simulink model "ClimaModMgr"  (R2022b)
settings: Solver=FixedStepDiscrete  StartTime=0.0  StopTime=10.0  FixedStep=0.1  RelTol=1e-3
settings Model: Creator=blue.ge  LastModifiedBy=blue.ge
settings DataIOCC: SaveFormat=StructureWithTime
settings OptimizationCC: DefaultParameterBehavior=Inlined  EnhancedBackFolding=on  PassReuseOutputArgsAs=Individual arguments  ZeroExternalMemoryAtStartup=off  ZeroInternalMemoryAtStartup=off  OptimizeBlockOrder=speed  DifferentSizesBufferReuse=on  OptimizationCustomize=off  EfficientTunableParamExpr=on
settings DebuggingCC: ParameterTunabilityLossMsg=error
settings ModelReferenceCC: ModelReferenceNumInstancesAllowed=Single
settings SFSimCC: MATLABDynamicMemAlloc=off
settings RTWCC: Description=AUTOSAR  SystemTargetFile=autosar.tlc  GenCodeOnly=on  TemplateMakefile=ert_default_tmf
settings CodeAppCC: ForceParamTrailComments=on  IgnoreCustomStorageClasses=off  OperatorAnnotations=on  SimulinkDataObjDesc=on  SFDataObjDesc=on  InsertBlockDesc=on
settings STFCustomTargetCC: TargetLangStandard=C99 (ISO)  MultiwordLength=256  ParMdlRefBuildCompliant=on  CompOptLevelCompliant=on  IncludeMdlTerminateFcn=off  CombineOutputUpdateFcns=on  SuppressErrorStatus=on  ERTCustomFileBanners=on  SupportNonFinite=off  SupportComplex=off  SupportContinuousTime=off  SupportNonInlinedSFcns=off  RemoveResetFunc=on  MATLABClassNameForMDSCustomization=Simulink.SoftwareTarget.ERTCustomization  AutosarCompliant=on  UseToolchainInfoCompliant=on  LookupTableObjectStructAxisOrder=2,1,3,4,...  LUTObjectStructOrderEvenSpacing=Size,Table,Breakpoints  SystemTargetFile=autosar.tlc
settings ERTTargetCC: TargetLangStandard=C99 (ISO)  ConcurrentExecutionCompliant=off  IncludeMdlTerminateFcn=off  SuppressErrorStatus=on  SupportNonFinite=off  SupportComplex=off  ModelStepFunctionPrototypeControlCompliant=off  CPPClassGenCompliant=off  AutosarCompliant=on  LookupTableObjectStructAxisOrder=2,1,3,4,...  LUTObjectStructOrderEvenSpacing=Size,Table,Breakpoints  InlinedParameterPlacement=NonHierarchical  ERTCustomFileTemplate=example_file_process.tlc

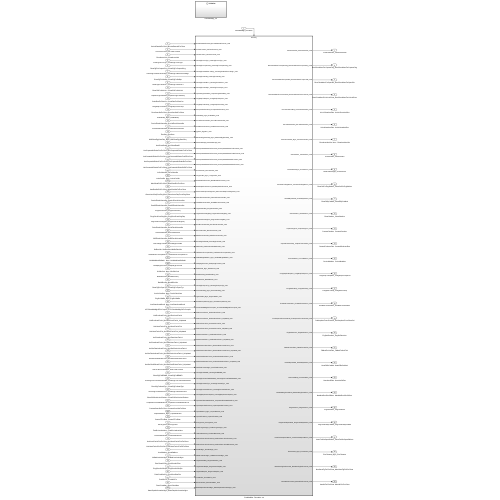
[thumbnail: Simulink's stored preview of the top-level diagram]
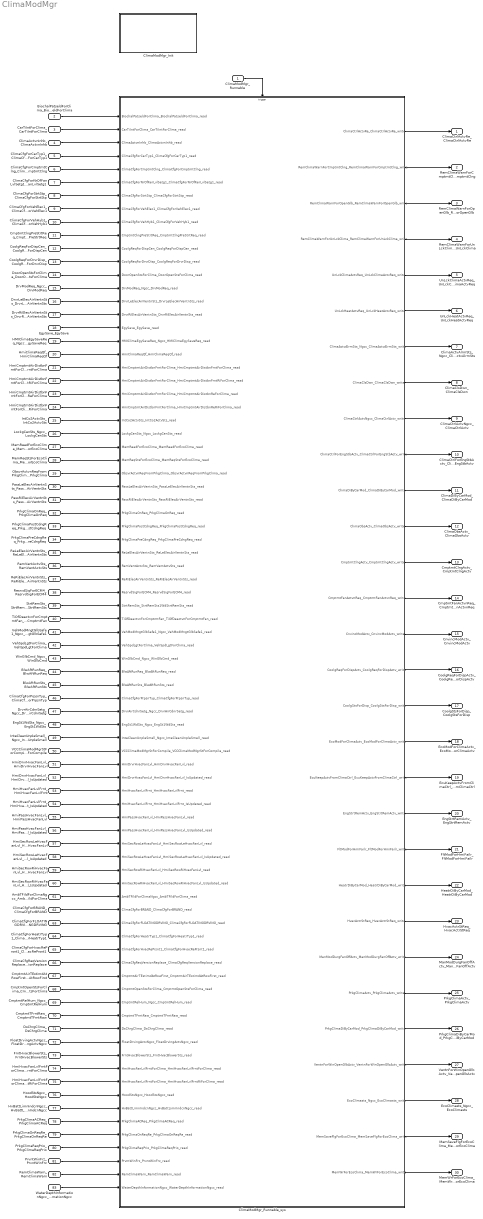
== system ClimaModMgr ==
  ClimaModMgr_Runnable : Inport  SampleTime=0.1  OutputFunctionCall=on
  BiochalPatseldForClima_BiochalPatseldForClima : Inport  Port=2  OutDataTypeStr=Boolean  PortDimensions=1
  CarTiIntForClima_CarTiIntForClima : Inport  Port=3  OutDataTypeStr=CarTiGlb  PortDimensions=1
  ClimaActvnInhb_ClimaActvnInhb : Inport  Port=4  OutDataTypeStr=Enum: OnOff1  PortDimensions=1
  ClimaCfgForCarTyp1_ClimaCfgForCarTyp1 : Inport  Port=5  OutDataTypeStr=Enum: ClimaCfgForCarTyp1  PortDimensions=1
  ClimaCfgForCmptmtClng_ClimaCfgForCmptmtClng : Inport  Port=6  OutDataTypeStr=Boolean  PortDimensions=1
  ClimaCfgForNrOfFanLvlSetg1_ClimaCfgForNrOfFanLvlSetg1 : Inport  Port=7  OutDataTypeStr=Enum: ClimaCfgForNrOfFanLvlSetg1  PortDimensions=1
  ClimaCfgForStrtStp_ClimaCfgForStrtStp : Inport  Port=8  OutDataTypeStr=Enum: CarCfgForStrtStop  PortDimensions=1
  ClimaCfgForVehElec1_ClimaCfgForVehElec1 : Inport  Port=9  OutDataTypeStr=Boolean  PortDimensions=1
  ClimaCfgForVehHyb1_ClimaCfgForVehHyb1 : Inport  Port=10  OutDataTypeStr=Boolean  PortDimensions=1
  CmptmtClngPreStrtReq_CmptmtClngPreStrtReq : Inport  Port=11  OutDataTypeStr=Enum: OnOffNoReq  PortDimensions=1
  CoolgReqForDispCen_CoolgReqForDispCen : Inport  Port=12  OutDataTypeStr=Enum: CoolgReqForDisp  PortDimensions=1
  CoolgReqForDrvrDisp_CoolgReqForDrvrDisp : Inport  Port=13  OutDataTypeStr=Enum: CoolgReqForDisp  PortDimensions=1
  DoorOpenStsForClima_DoorOpenStsForClima : Inport  Port=14  OutDataTypeStr=Boolean  PortDimensions=1
  DrvModReq_Ngcc_DrvModReq : Inport  Port=15  OutDataTypeStr=Enum: DrvModReqType1  PortDimensions=1
  DrvrLeElecAirVentnSts_DrvrLeElecAirVentnSts : Inport  Port=16  OutDataTypeStr=Enum: OnOff1  PortDimensions=1
  DrvrRiElecAirVentnSts_DrvrRiElecAirVentnSts : Inport  Port=17  OutDataTypeStr=Enum: OnOff1  PortDimensions=1
  EgySave_EgySave : Inport  Port=18  OutDataTypeStr=Enum: OnOff1  PortDimensions=1
  HMIClimaEgySaveReq_Ngcc_HMIClimaEgySaveReq : Inport  Port=19  OutDataTypeStr=Enum: VentnActr01BlckInd  PortDimensions=1
  HmiClimaReqQf_HmiClimaReqQf : Inport  Port=20  OutDataTypeStr=Enum: HmiClimaReqQf  PortDimensions=1
  HmiCmptmtAirDistbnFrntForClima_HmiCmptmtAirDistbnFrntForClima : Inport  Port=21  OutDataTypeStr=Enum: HmiCmptmtAirDistbnFrnt  PortDimensions=1
  HmiCmptmtAirDistbnFrntForClima_HmiCmptmtAirDistbnFrntRiForClima : Inport  Port=22  OutDataTypeStr=Enum: HmiCmptmtAirDistbnFrnt  PortDimensions=1
  HmiCmptmtAirDistbnFrntForClima_HmiCmptmtAirDistbnReForClima : Inport  Port=23  OutDataTypeStr=Enum: HmiCmptmtAirDistbnFrnt  PortDimensions=1
  HmiCmptmtAirDistbnFrntForClima_HmiCmptmtAirDistbnReRiForClima : Inport  Port=24  OutDataTypeStr=Enum: HmiCmptmtAirDistbnFrnt  PortDimensions=1
  IntCo2ActvSts_IntCo2ActvSts : Inport  Port=25  OutDataTypeStr=Enum: IntCo2Sts  PortDimensions=1
  LockgCenSts_Ngcc_LockgCenSts : Inport  Port=26  OutDataTypeStr=Bus: LockgCenSts3  PortDimensions=1  BusOutputAsStruct=on
  MemReadForEcoClima_MemReadForEcoClima : Inport  Port=27  OutDataTypeStr=Bus: MemWrForEcoClima  PortDimensions=1  BusOutputAsStruct=on
  MemReqStsForEcoClima_MemReqStsForEcoClima : Inport  Port=28  OutDataTypeStr=Enum: MemReqSts  PortDimensions=1
  ObsvrActvnReqFromPrkgClima_ObsvrActvnReqFromPrkgClima : Inport  Port=29  OutDataTypeStr=Boolean  PortDimensions=1
  PassLeElecAirVentnSts_PassLeElecAirVentnSts : Inport  Port=30  OutDataTypeStr=Enum: OnOff1  PortDimensions=1
  PassRiElecAirVentnSts_PassRiElecAirVentnSts : Inport  Port=31  OutDataTypeStr=Enum: OnOff1  PortDimensions=1
  PrkgClimaOnReq_PrkgClimaOnReq : Inport  Port=32  OutDataTypeStr=Boolean  PortDimensions=1
  PrkgClimaPostCdngReq_PrkgClimaPostCdngReq : Inport  Port=33  OutDataTypeStr=Boolean  PortDimensions=1
  PrkgClimaPreCdngReq_PrkgClimaPreCdngReq : Inport  Port=34  OutDataTypeStr=Boolean  PortDimensions=1
  ReLeElecAirVentnSts_ReLeElecAirVentnSts : Inport  Port=35  OutDataTypeStr=Enum: OnOff1  PortDimensions=1
  RemVentActvSts_RemVentActvSts : Inport  Port=36  OutDataTypeStr=Enum: OnOff1  PortDimensions=1
  ReRiElecAirVentnSts_ReRiElecAirVentnSts : Inport  Port=37  OutDataTypeStr=Enum: OnOff1  PortDimensions=1
  ResrvdSigForECM4_ResrvdSigForECM4 : Inport  Port=38  OutDataTypeStr=BattSnsrChksTbl  PortDimensions=1
  StrtRemSts_StrtRemSts1WdStrtRemSts : Inport  Port=39  OutDataTypeStr=Enum: StrtRemSts1  PortDimensions=1
  TiOfDeactvnForCmptmtFan_TiOfDeactvnForCmptmtFan : Inport  Port=40  OutDataTypeStr=Ti33  PortDimensions=1
  VehModMngtGlbSafe1_Ngcc_VehModMngtGlbSafe1 : Inport  Port=41  OutDataTypeStr=Bus: VehModMngtGlbSafe1  PortDimensions=1  BusOutputAsStruct=on
  VehSpdLgtForClima_VehSpdLgtForClima : Inport  Port=42  OutDataTypeStr=Bus: VehSpdLgtForClima  PortDimensions=1  BusOutputAsStruct=on
  WinGlbCmd_Ngcc_WinGlbCmd : Inport  Port=43  OutDataTypeStr=Enum: OpenClsGlbCmd  PortDimensions=1
  BlwAftRunReq_BlwAftRunReq : Inport  Port=44  OutDataTypeStr=Boolean  PortDimensions=1
  BlwAftRunSts_BlwAftRunSts : Inport  Port=45  OutDataTypeStr=Boolean  PortDimensions=1
  ClimaCfgForPrpsnTyp_ClimaCfgForPrpsnTyp : Inport  Port=46  OutDataTypeStr=Enum: CarCfgForPrpsnTypGly  PortDimensions=1
  DrvrAirCdnrSetg_Ngcc_DrvrAirCdnrSetg : Inport  Port=47  OutDataTypeStr=Bus: DrvrAirCdnrSetg  PortDimensions=1  BusOutputAsStruct=on
  EngSt1WdSts_Ngcc_EngSt1WdSts : Inport  Port=48  OutDataTypeStr=Enum: EngSt1  PortDimensions=1
  InteCleanUnpleSmell_Ngcc_InteCleanUnpleSmell : Inport  Port=49  OutDataTypeStr=Enum: OnOff1  PortDimensions=1
  VCCClimaModMgrStForCompile_VCCClimaModMgrStForCompile : Inport  Port=50  OutDataTypeStr=Enum: VCCClimaModMgrSt  PortDimensions=1
  HmiDrvrHvacFanLvl_HmiDrvrHvacFanLvl : Inport  Port=51  OutDataTypeStr=Enum: HmiHvacFanLvl  PortDimensions=1
  HmiDrvrHvacFanLvl_HmiDrvrHvacFanLvl_IsUpdated : Inport  Port=52  OutDataTypeStr=boolean  PortDimensions=1
  HmiHvacFanLvlFrnt_HmiHvacFanLvlFrnt : Inport  Port=53  OutDataTypeStr=Enum: HmiHvacFanLvl  PortDimensions=1
  HmiHvacFanLvlFrnt_HmiHvacFanLvlFrnt_IsUpdated : Inport  Port=54  OutDataTypeStr=boolean  PortDimensions=1
  HmiPassHvacFanLvl_HmiPassHvacFanLvl : Inport  Port=55  OutDataTypeStr=Enum: HmiHvacFanLvl  PortDimensions=1
  HmiPassHvacFanLvl_HmiPassHvacFanLvl_IsUpdated : Inport  Port=56  OutDataTypeStr=boolean  PortDimensions=1
  HmiSecRowLeHvacFanLvl_HmiSecRowLeHvacFanLvl : Inport  Port=57  OutDataTypeStr=Enum: HmiHvacFanLvl  PortDimensions=1
  HmiSecRowLeHvacFanLvl_HmiSecRowLeHvacFanLvl_IsUpdated : Inport  Port=58  OutDataTypeStr=boolean  PortDimensions=1
  HmiSecRowRiHvacFanLvl_HmiSecRowRiHvacFanLvl : Inport  Port=59  OutDataTypeStr=Enum: HmiHvacFanLvl  PortDimensions=1
  HmiSecRowRiHvacFanLvl_HmiSecRowRiHvacFanLvl_IsUpdated : Inport  Port=60  OutDataTypeStr=boolean  PortDimensions=1
  AmbTFildForClimaNgcc_AmbTFildForClima : Inport  Port=61  OutDataTypeStr=T30  PortDimensions=1
  ClimaCfgForBRAND_ClimaCfgForBRAND : Inport  Port=62  OutDataTypeStr=Enum: ClimaCfgForBRAND  PortDimensions=1
  ClimaCfgForFLOATINGDRVING_ClimaCfgForFLOATINGDRVING : Inport  Port=63  OutDataTypeStr=Enum: FLOATINGDRVING  PortDimensions=1
  ClimaCfgForHeatrTyp1_ClimaCfgForHeatrTyp1 : Inport  Port=64  OutDataTypeStr=Enum: ClimaCfgForHeatrTyp1  PortDimensions=1
  ClimaCfgForHvacRePrsnt1_ClimaCfgForHvacRePrsnt1 : Inport  Port=65  OutDataTypeStr=Enum: CarCfgForHvacRe  PortDimensions=1
  ClimaCfgReqVersionReplace_ClimaCfgReqVersionReplace : Inport  Port=66  OutDataTypeStr=UInt8  PortDimensions=1
  CmptmtAirTEstimdAtRowFirst_CmptmtAirTEstimdAtRowFirst : Inport  Port=67  OutDataTypeStr=T30  PortDimensions=1
  CmptmtOpenStsForClima_CmptmtOpenStsForClima : Inport  Port=68  OutDataTypeStr=Boolean  PortDimensions=1
  CmptmtRelHum_Ngcc_CmptmtRelHum : Inport  Port=69  OutDataTypeStr=Bus: CmptmRelHum  PortDimensions=1  BusOutputAsStruct=on
  CmptmtTFrntRaw_CmptmtTFrntRaw : Inport  Port=70  OutDataTypeStr=Bus: CmptmtTFrnt  PortDimensions=1  BusOutputAsStruct=on
  DsChrgClima_DsChrgClima : Inport  Port=71  OutDataTypeStr=Boolean  PortDimensions=1
  FloatDrvingActvNgcc_FloatDrvingActvNgcc : Inport  Port=72  OutDataTypeStr=Bus: FloatDrvingActv  PortDimensions=1  BusOutputAsStruct=on
  FrntHvacBlowerSts_FrntHvacBlowerSts : Inport  Port=73  OutDataTypeStr=Enum: HvacFanSts  PortDimensions=1
  HmiHvacFanLvlFrntForClima_HmiHvacFanLvlFrntForClima : Inport  Port=74  OutDataTypeStr=Enum: HmiHvacFanLvl  PortDimensions=1
  HmiHvacFanLvlFrntForClima_HmiHvacFanLvlFrntRiForClima : Inport  Port=75  OutDataTypeStr=Enum: HmiHvacFanLvl  PortDimensions=1
  HoodStsNgcc_HoodStsNgcc : Inport  Port=76  OutDataTypeStr=Enum: DoorSts2  PortDimensions=1
  HvBattLimnIndcnNgcc_HvBattLimnIndcnNgcc : Inport  Port=77  OutDataTypeStr=UInt16  PortDimensions=1
  PrkgClimaACReq_PrkgClimaACReq : Inport  Port=78  OutDataTypeStr=Enum: HmiCmptmtCoolgReq  PortDimensions=1
  PrkgClimaOnReqRe_PrkgClimaOnReqRe : Inport  Port=79  OutDataTypeStr=Enum: OnOff1  PortDimensions=1
  PrkgClimaReqPrio_PrkgClimaReqPrio : Inport  Port=80  OutDataTypeStr=Boolean  PortDimensions=1
  PrvntWinFrz_PrvntWinFrz : Inport  Port=81  OutDataTypeStr=Boolean  PortDimensions=1
  RemClimaWarn_RemClimaWarn : Inport  Port=82  OutDataTypeStr=Enum: ClimaWarn  PortDimensions=1
  WaterDepthInformationNgcc_WaterDepthInformationNgcc : Inport  Port=83  OutDataTypeStr=Bus: WaterDepthInformation  PortDimensions=1  BusOutputAsStruct=on
  ClimaModMgr_Init : Subsystem
  ClimaModMgr_Runnable_sys : Subsystem
  ClimaCtrlActvRe_ClimaCtrlActvRe : Outport  OutDataTypeStr=Boolean  PortDimensions=1
  RemClimaWarnForCmptmtClng_RemClimaWarnForCmptmtClng : Outport  Port=2  OutDataTypeStr=Enum: ClimaWarn  PortDimensions=1
  RemClimaWarnForOpenGlb_RemClimaWarnForOpenGlb : Outport  Port=3  OutDataTypeStr=Enum: ClimaWarn  PortDimensions=1
  RemClimaWarnForUnLckClima_RemClimaWarnForUnLckClima : Outport  Port=4  OutDataTypeStr=Enum: ClimaWarn  PortDimensions=1
  UnLckClimaActvReq_UnLckClimaActvReq : Outport  Port=5  OutDataTypeStr=Enum: UnLckCoolActvLvl  PortDimensions=1
  UnLckHeatActvReq_UnLckHeatActvReq : Outport  Port=6  OutDataTypeStr=Enum: UnLckHeatActvLvl  PortDimensions=1
  ClimaActvAlrmSts_Ngcc_ClimaActvAlrmSts : Outport  Port=7  OutDataTypeStr=Enum: OnOff1  PortDimensions=1
  ClimaClsDwn_ClimaClsDwn : Outport  Port=8  OutDataTypeStr=Boolean  PortDimensions=1
  ClimaCtrlActvNgcc_ClimaCtrlActv : Outport  Port=9  OutDataTypeStr=Boolean  PortDimensions=1
  ClimaCtrlForEngStbActv_ClimaCtrlForEngStbActv : Outport  Port=10  OutDataTypeStr=Boolean  PortDimensions=1
  ClimaDiByCarMod_ClimaDiByCarMod : Outport  Port=11  OutDataTypeStr=Boolean  PortDimensions=1
  ClimaObsActv_ClimaObsActv : Outport  Port=12  OutDataTypeStr=Boolean  PortDimensions=1
  CmptmtClngActv_CmptmtClngActv : Outport  Port=13  OutDataTypeStr=Enum: CmptmtClngActv  PortDimensions=1
  CmptmtFanActvnReq_CmptmtFanActvnReq : Outport  Port=14  OutDataTypeStr=Enum: OnOff1  PortDimensions=1
  CnvincModActv_CnvincModActv : Outport  Port=15  OutDataTypeStr=Boolean  PortDimensions=1
  CoolgReqForDispActv_CoolgReqForDispActv : Outport  Port=16  OutDataTypeStr=Enum: CoolgReqForDisp  PortDimensions=1
  CoolgStsForDisp_CoolgStsForDisp : Outport  Port=17  OutDataTypeStr=Enum: CoolgStsForDisp  PortDimensions=1
  EcoModForClimaActv_EcoModForClimaActv : Outport  Port=18  OutDataTypeStr=Enum: OnOff1  PortDimensions=1
  EcuKeepActvFromClimaCtrl_EcuKeepActvFromClimaCtrl : Outport  Port=19  OutDataTypeStr=Enum: OnOff1  PortDimensions=1
  EngStrtRemActv_EngStrtRemActv : Outport  Port=20  OutDataTypeStr=Boolean  PortDimensions=1
  FltModForHmiFailr_FltModForHmiFailr : Outport  Port=21  OutDataTypeStr=Boolean  PortDimensions=1
  HeatrDiByCarMod_HeatrDiByCarMod : Outport  Port=22  OutDataTypeStr=Boolean  PortDimensions=1
  HvacActrStReq_HvacActrStReq : Outport  Port=23  OutDataTypeStr=Enum: HvacActrSt  PortDimensions=1
  ManModDurgFanOffActv_ManModDurgFanOffActv : Outport  Port=24  OutDataTypeStr=Boolean  PortDimensions=1
  PrkgClimaActv_PrkgClimaActv : Outport  Port=25  OutDataTypeStr=Enum: PrkgClimaActv  PortDimensions=1
  PrkgClimaDiByCarMod_PrkgClimaDiByCarMod : Outport  Port=26  OutDataTypeStr=Boolean  PortDimensions=1
  VentnForWinOpenGlbActv_VentnForWinOpenGlbActv : Outport  Port=27  OutDataTypeStr=Boolean  PortDimensions=1
  EcoClimasts_Ngcc_EcoClimasts : Outport  Port=28  OutDataTypeStr=Enum: OnOff1  PortDimensions=1
  MemSaveFlgForEcoClima_MemSaveFlgForEcoClima : Outport  Port=29  OutDataTypeStr=Boolean  PortDimensions=1
  MemWrForEcoClima_MemWrForEcoClima : Outport  Port=30  OutDataTypeStr=Bus: MemWrForEcoClima  PortDimensions=1  BusOutputAsStruct=on
  wires:
  BiochalPatseldForClima_BiochalPatseldForClima/1 -> ClimaModMgr_Runnable_sys/1(BiochalPatseldForClima_BiochalPatseldForClima_read)
  CarTiIntForClima_CarTiIntForClima/1 -> ClimaModMgr_Runnable_sys/2(CarTiIntForClima_CarTiIntForClima_read)
  ClimaActvnInhb_ClimaActvnInhb/1 -> ClimaModMgr_Runnable_sys/3(ClimaActvnInhb_ClimaActvnInhb_read)
  ClimaCfgForCarTyp1_ClimaCfgForCarTyp1/1 -> ClimaModMgr_Runnable_sys/4(ClimaCfgForCarTyp1_ClimaCfgForCarTyp1_read)
  ClimaCfgForCmptmtClng_ClimaCfgForCmptmtClng/1 -> ClimaModMgr_Runnable_sys/5(ClimaCfgForCmptmtClng_ClimaCfgForCmptmtClng_read)
  ClimaCfgForNrOfFanLvlSetg1_ClimaCfgForNrOfFanLvlSetg1/1 -> ClimaModMgr_Runnable_sys/6(ClimaCfgForNrOfFanLvlSetg1_ClimaCfgForNrOfFanLvlSetg1_read)
  ClimaCfgForStrtStp_ClimaCfgForStrtStp/1 -> ClimaModMgr_Runnable_sys/7(ClimaCfgForStrtStp_ClimaCfgForStrtStp_read)
  ClimaCfgForVehElec1_ClimaCfgForVehElec1/1 -> ClimaModMgr_Runnable_sys/8(ClimaCfgForVehElec1_ClimaCfgForVehElec1_read)
  ClimaCfgForVehHyb1_ClimaCfgForVehHyb1/1 -> ClimaModMgr_Runnable_sys/9(ClimaCfgForVehHyb1_ClimaCfgForVehHyb1_read)
  CmptmtClngPreStrtReq_CmptmtClngPreStrtReq/1 -> ClimaModMgr_Runnable_sys/10(CmptmtClngPreStrtReq_CmptmtClngPreStrtReq_read)
  CoolgReqForDispCen_CoolgReqForDispCen/1 -> ClimaModMgr_Runnable_sys/11(CoolgReqForDispCen_CoolgReqForDispCen_read)
  CoolgReqForDrvrDisp_CoolgReqForDrvrDisp/1 -> ClimaModMgr_Runnable_sys/12(CoolgReqForDrvrDisp_CoolgReqForDrvrDisp_read)
  DoorOpenStsForClima_DoorOpenStsForClima/1 -> ClimaModMgr_Runnable_sys/13(DoorOpenStsForClima_DoorOpenStsForClima_read)
  DrvModReq_Ngcc_DrvModReq/1 -> ClimaModMgr_Runnable_sys/14(DrvModReq_Ngcc_DrvModReq_read)
  DrvrLeElecAirVentnSts_DrvrLeElecAirVentnSts/1 -> ClimaModMgr_Runnable_sys/15(DrvrLeElecAirVentnSts_DrvrLeElecAirVentnSts_read)
  DrvrRiElecAirVentnSts_DrvrRiElecAirVentnSts/1 -> ClimaModMgr_Runnable_sys/16(DrvrRiElecAirVentnSts_DrvrRiElecAirVentnSts_read)
  EgySave_EgySave/1 -> ClimaModMgr_Runnable_sys/17(EgySave_EgySave_read)
  HMIClimaEgySaveReq_Ngcc_HMIClimaEgySaveReq/1 -> ClimaModMgr_Runnable_sys/18(HMIClimaEgySaveReq_Ngcc_HMIClimaEgySaveReq_read)
  HmiClimaReqQf_HmiClimaReqQf/1 -> ClimaModMgr_Runnable_sys/19(HmiClimaReqQf_HmiClimaReqQf_read)
  HmiCmptmtAirDistbnFrntForClima_HmiCmptmtAirDistbnFrntForClima/1 -> ClimaModMgr_Runnable_sys/20(HmiCmptmtAirDistbnFrntForClima_HmiCmptmtAirDistbnFrntForClima_read)
  HmiCmptmtAirDistbnFrntForClima_HmiCmptmtAirDistbnFrntRiForClima/1 -> ClimaModMgr_Runnable_sys/21(HmiCmptmtAirDistbnFrntForClima_HmiCmptmtAirDistbnFrntRiForClima_read)
  HmiCmptmtAirDistbnFrntForClima_HmiCmptmtAirDistbnReForClima/1 -> ClimaModMgr_Runnable_sys/22(HmiCmptmtAirDistbnFrntForClima_HmiCmptmtAirDistbnReForClima_read)
  HmiCmptmtAirDistbnFrntForClima_HmiCmptmtAirDistbnReRiForClima/1 -> ClimaModMgr_Runnable_sys/23(HmiCmptmtAirDistbnFrntForClima_HmiCmptmtAirDistbnReRiForClima_read)
  IntCo2ActvSts_IntCo2ActvSts/1 -> ClimaModMgr_Runnable_sys/24(IntCo2ActvSts_IntCo2ActvSts_read)
  LockgCenSts_Ngcc_LockgCenSts/1 -> ClimaModMgr_Runnable_sys/25(LockgCenSts_Ngcc_LockgCenSts_read)
  MemReadForEcoClima_MemReadForEcoClima/1 -> ClimaModMgr_Runnable_sys/26(MemReadForEcoClima_MemReadForEcoClima_read)
  MemReqStsForEcoClima_MemReqStsForEcoClima/1 -> ClimaModMgr_Runnable_sys/27(MemReqStsForEcoClima_MemReqStsForEcoClima_read)
  ObsvrActvnReqFromPrkgClima_ObsvrActvnReqFromPrkgClima/1 -> ClimaModMgr_Runnable_sys/28(ObsvrActvnReqFromPrkgClima_ObsvrActvnReqFromPrkgClima_read)
  PassLeElecAirVentnSts_PassLeElecAirVentnSts/1 -> ClimaModMgr_Runnable_sys/29(PassLeElecAirVentnSts_PassLeElecAirVentnSts_read)
  PassRiElecAirVentnSts_PassRiElecAirVentnSts/1 -> ClimaModMgr_Runnable_sys/30(PassRiElecAirVentnSts_PassRiElecAirVentnSts_read)
  PrkgClimaOnReq_PrkgClimaOnReq/1 -> ClimaModMgr_Runnable_sys/31(PrkgClimaOnReq_PrkgClimaOnReq_read)
  PrkgClimaPostCdngReq_PrkgClimaPostCdngReq/1 -> ClimaModMgr_Runnable_sys/32(PrkgClimaPostCdngReq_PrkgClimaPostCdngReq_read)
  PrkgClimaPreCdngReq_PrkgClimaPreCdngReq/1 -> ClimaModMgr_Runnable_sys/33(PrkgClimaPreCdngReq_PrkgClimaPreCdngReq_read)
  ReLeElecAirVentnSts_ReLeElecAirVentnSts/1 -> ClimaModMgr_Runnable_sys/34(ReLeElecAirVentnSts_ReLeElecAirVentnSts_read)
  RemVentActvSts_RemVentActvSts/1 -> ClimaModMgr_Runnable_sys/35(RemVentActvSts_RemVentActvSts_read)
  ReRiElecAirVentnSts_ReRiElecAirVentnSts/1 -> ClimaModMgr_Runnable_sys/36(ReRiElecAirVentnSts_ReRiElecAirVentnSts_read)
  ResrvdSigForECM4_ResrvdSigForECM4/1 -> ClimaModMgr_Runnable_sys/37(ResrvdSigForECM4_ResrvdSigForECM4_read)
  StrtRemSts_StrtRemSts1WdStrtRemSts/1 -> ClimaModMgr_Runnable_sys/38(StrtRemSts_StrtRemSts1WdStrtRemSts_read)
  TiOfDeactvnForCmptmtFan_TiOfDeactvnForCmptmtFan/1 -> ClimaModMgr_Runnable_sys/39(TiOfDeactvnForCmptmtFan_TiOfDeactvnForCmptmtFan_read)
  VehModMngtGlbSafe1_Ngcc_VehModMngtGlbSafe1/1 -> ClimaModMgr_Runnable_sys/40(VehModMngtGlbSafe1_Ngcc_VehModMngtGlbSafe1_read)
  VehSpdLgtForClima_VehSpdLgtForClima/1 -> ClimaModMgr_Runnable_sys/41(VehSpdLgtForClima_VehSpdLgtForClima_read)
  WinGlbCmd_Ngcc_WinGlbCmd/1 -> ClimaModMgr_Runnable_sys/42(WinGlbCmd_Ngcc_WinGlbCmd_read)
  BlwAftRunReq_BlwAftRunReq/1 -> ClimaModMgr_Runnable_sys/43(BlwAftRunReq_BlwAftRunReq_read)
  BlwAftRunSts_BlwAftRunSts/1 -> ClimaModMgr_Runnable_sys/44(BlwAftRunSts_BlwAftRunSts_read)
  ClimaCfgForPrpsnTyp_ClimaCfgForPrpsnTyp/1 -> ClimaModMgr_Runnable_sys/45(ClimaCfgForPrpsnTyp_ClimaCfgForPrpsnTyp_read)
  DrvrAirCdnrSetg_Ngcc_DrvrAirCdnrSetg/1 -> ClimaModMgr_Runnable_sys/46(DrvrAirCdnrSetg_Ngcc_DrvrAirCdnrSetg_read)
  EngSt1WdSts_Ngcc_EngSt1WdSts/1 -> ClimaModMgr_Runnable_sys/47(EngSt1WdSts_Ngcc_EngSt1WdSts_read)
  InteCleanUnpleSmell_Ngcc_InteCleanUnpleSmell/1 -> ClimaModMgr_Runnable_sys/48(InteCleanUnpleSmell_Ngcc_InteCleanUnpleSmell_read)
  VCCClimaModMgrStForCompile_VCCClimaModMgrStForCompile/1 -> ClimaModMgr_Runnable_sys/49(VCCClimaModMgrStForCompile_VCCClimaModMgrStForCompile_read)
  HmiDrvrHvacFanLvl_HmiDrvrHvacFanLvl/1 -> ClimaModMgr_Runnable_sys/50(HmiDrvrHvacFanLvl_HmiDrvrHvacFanLvl_read)
  HmiDrvrHvacFanLvl_HmiDrvrHvacFanLvl_IsUpdated/1 -> ClimaModMgr_Runnable_sys/51(HmiDrvrHvacFanLvl_HmiDrvrHvacFanLvl_IsUpdated_read)
  HmiHvacFanLvlFrnt_HmiHvacFanLvlFrnt/1 -> ClimaModMgr_Runnable_sys/52(HmiHvacFanLvlFrnt_HmiHvacFanLvlFrnt_read)
  HmiHvacFanLvlFrnt_HmiHvacFanLvlFrnt_IsUpdated/1 -> ClimaModMgr_Runnable_sys/53(HmiHvacFanLvlFrnt_HmiHvacFanLvlFrnt_IsUpdated_read)
  HmiPassHvacFanLvl_HmiPassHvacFanLvl/1 -> ClimaModMgr_Runnable_sys/54(HmiPassHvacFanLvl_HmiPassHvacFanLvl_read)
  HmiPassHvacFanLvl_HmiPassHvacFanLvl_IsUpdated/1 -> ClimaModMgr_Runnable_sys/55(HmiPassHvacFanLvl_HmiPassHvacFanLvl_IsUpdated_read)
  HmiSecRowLeHvacFanLvl_HmiSecRowLeHvacFanLvl/1 -> ClimaModMgr_Runnable_sys/56(HmiSecRowLeHvacFanLvl_HmiSecRowLeHvacFanLvl_read)
  HmiSecRowLeHvacFanLvl_HmiSecRowLeHvacFanLvl_IsUpdated/1 -> ClimaModMgr_Runnable_sys/57(HmiSecRowLeHvacFanLvl_HmiSecRowLeHvacFanLvl_IsUpdated_read)
  HmiSecRowRiHvacFanLvl_HmiSecRowRiHvacFanLvl/1 -> ClimaModMgr_Runnable_sys/58(HmiSecRowRiHvacFanLvl_HmiSecRowRiHvacFanLvl_read)
  HmiSecRowRiHvacFanLvl_HmiSecRowRiHvacFanLvl_IsUpdated/1 -> ClimaModMgr_Runnable_sys/59(HmiSecRowRiHvacFanLvl_HmiSecRowRiHvacFanLvl_IsUpdated_read)
  AmbTFildForClimaNgcc_AmbTFildForClima/1 -> ClimaModMgr_Runnable_sys/60(AmbTFildForClimaNgcc_AmbTFildForClima_read)
  ClimaCfgForBRAND_ClimaCfgForBRAND/1 -> ClimaModMgr_Runnable_sys/61(ClimaCfgForBRAND_ClimaCfgForBRAND_read)
  ClimaCfgForFLOATINGDRVING_ClimaCfgForFLOATINGDRVING/1 -> ClimaModMgr_Runnable_sys/62(ClimaCfgForFLOATINGDRVING_ClimaCfgForFLOATINGDRVING_read)
  ClimaCfgForHeatrTyp1_ClimaCfgForHeatrTyp1/1 -> ClimaModMgr_Runnable_sys/63(ClimaCfgForHeatrTyp1_ClimaCfgForHeatrTyp1_read)
  ClimaCfgForHvacRePrsnt1_ClimaCfgForHvacRePrsnt1/1 -> ClimaModMgr_Runnable_sys/64(ClimaCfgForHvacRePrsnt1_ClimaCfgForHvacRePrsnt1_read)
  ClimaCfgReqVersionReplace_ClimaCfgReqVersionReplace/1 -> ClimaModMgr_Runnable_sys/65(ClimaCfgReqVersionReplace_ClimaCfgReqVersionReplace_read)
  CmptmtAirTEstimdAtRowFirst_CmptmtAirTEstimdAtRowFirst/1 -> ClimaModMgr_Runnable_sys/66(CmptmtAirTEstimdAtRowFirst_CmptmtAirTEstimdAtRowFirst_read)
  CmptmtOpenStsForClima_CmptmtOpenStsForClima/1 -> ClimaModMgr_Runnable_sys/67(CmptmtOpenStsForClima_CmptmtOpenStsForClima_read)
  CmptmtRelHum_Ngcc_CmptmtRelHum/1 -> ClimaModMgr_Runnable_sys/68(CmptmtRelHum_Ngcc_CmptmtRelHum_read)
  CmptmtTFrntRaw_CmptmtTFrntRaw/1 -> ClimaModMgr_Runnable_sys/69(CmptmtTFrntRaw_CmptmtTFrntRaw_read)
  DsChrgClima_DsChrgClima/1 -> ClimaModMgr_Runnable_sys/70(DsChrgClima_DsChrgClima_read)
  FloatDrvingActvNgcc_FloatDrvingActvNgcc/1 -> ClimaModMgr_Runnable_sys/71(FloatDrvingActvNgcc_FloatDrvingActvNgcc_read)
  FrntHvacBlowerSts_FrntHvacBlowerSts/1 -> ClimaModMgr_Runnable_sys/72(FrntHvacBlowerSts_FrntHvacBlowerSts_read)
  HmiHvacFanLvlFrntForClima_HmiHvacFanLvlFrntForClima/1 -> ClimaModMgr_Runnable_sys/73(HmiHvacFanLvlFrntForClima_HmiHvacFanLvlFrntForClima_read)
  HmiHvacFanLvlFrntForClima_HmiHvacFanLvlFrntRiForClima/1 -> ClimaModMgr_Runnable_sys/74(HmiHvacFanLvlFrntForClima_HmiHvacFanLvlFrntRiForClima_read)
  HoodStsNgcc_HoodStsNgcc/1 -> ClimaModMgr_Runnable_sys/75(HoodStsNgcc_HoodStsNgcc_read)
  HvBattLimnIndcnNgcc_HvBattLimnIndcnNgcc/1 -> ClimaModMgr_Runnable_sys/76(HvBattLimnIndcnNgcc_HvBattLimnIndcnNgcc_read)
  PrkgClimaACReq_PrkgClimaACReq/1 -> ClimaModMgr_Runnable_sys/77(PrkgClimaACReq_PrkgClimaACReq_read)
  PrkgClimaOnReqRe_PrkgClimaOnReqRe/1 -> ClimaModMgr_Runnable_sys/78(PrkgClimaOnReqRe_PrkgClimaOnReqRe_read)
  PrkgClimaReqPrio_PrkgClimaReqPrio/1 -> ClimaModMgr_Runnable_sys/79(PrkgClimaReqPrio_PrkgClimaReqPrio_read)
  PrvntWinFrz_PrvntWinFrz/1 -> ClimaModMgr_Runnable_sys/80(PrvntWinFrz_PrvntWinFrz_read)
  RemClimaWarn_RemClimaWarn/1 -> ClimaModMgr_Runnable_sys/81(RemClimaWarn_RemClimaWarn_read)
  WaterDepthInformationNgcc_WaterDepthInformationNgcc/1 -> ClimaModMgr_Runnable_sys/82(WaterDepthInformationNgcc_WaterDepthInformationNgcc_read)
  ClimaModMgr_Runnable_sys/1(ClimaCtrlActvRe_ClimaCtrlActvRe_write) -> ClimaCtrlActvRe_ClimaCtrlActvRe/1
  ClimaModMgr_Runnable_sys/2(RemClimaWarnForCmptmtClng_RemClimaWarnForCmptmtClng_write) -> RemClimaWarnForCmptmtClng_RemClimaWarnForCmptmtClng/1
  ClimaModMgr_Runnable_sys/3(RemClimaWarnForOpenGlb_RemClimaWarnForOpenGlb_write) -> RemClimaWarnForOpenGlb_RemClimaWarnForOpenGlb/1
  ClimaModMgr_Runnable_sys/4(RemClimaWarnForUnLckClima_RemClimaWarnForUnLckClima_write) -> RemClimaWarnForUnLckClima_RemClimaWarnForUnLckClima/1
  ClimaModMgr_Runnable_sys/5(UnLckClimaActvReq_UnLckClimaActvReq_write) -> UnLckClimaActvReq_UnLckClimaActvReq/1
  ClimaModMgr_Runnable_sys/6(UnLckHeatActvReq_UnLckHeatActvReq_write) -> UnLckHeatActvReq_UnLckHeatActvReq/1
  ClimaModMgr_Runnable_sys/7(ClimaActvAlrmSts_Ngcc_ClimaActvAlrmSts_write) -> ClimaActvAlrmSts_Ngcc_ClimaActvAlrmSts/1
  ClimaModMgr_Runnable_sys/8(ClimaClsDwn_ClimaClsDwn_write) -> ClimaClsDwn_ClimaClsDwn/1
  ClimaModMgr_Runnable_sys/9(ClimaCtrlActvNgcc_ClimaCtrlActv_write) -> ClimaCtrlActvNgcc_ClimaCtrlActv/1
  ClimaModMgr_Runnable_sys/10(ClimaCtrlForEngStbActv_ClimaCtrlForEngStbActv_write) -> ClimaCtrlForEngStbActv_ClimaCtrlForEngStbActv/1
  ClimaModMgr_Runnable_sys/11(ClimaDiByCarMod_ClimaDiByCarMod_write) -> ClimaDiByCarMod_ClimaDiByCarMod/1
  ClimaModMgr_Runnable_sys/12(ClimaObsActv_ClimaObsActv_write) -> ClimaObsActv_ClimaObsActv/1
  ClimaModMgr_Runnable_sys/13(CmptmtClngActv_CmptmtClngActv_write) -> CmptmtClngActv_CmptmtClngActv/1
  ClimaModMgr_Runnable_sys/14(CmptmtFanActvnReq_CmptmtFanActvnReq_write) -> CmptmtFanActvnReq_CmptmtFanActvnReq/1
  ClimaModMgr_Runnable_sys/15(CnvincModActv_CnvincModActv_write) -> CnvincModActv_CnvincModActv/1
  ClimaModMgr_Runnable_sys/16(CoolgReqForDispActv_CoolgReqForDispActv_write) -> CoolgReqForDispActv_CoolgReqForDispActv/1
  ClimaModMgr_Runnable_sys/17(CoolgStsForDisp_CoolgStsForDisp_write) -> CoolgStsForDisp_CoolgStsForDisp/1
  ClimaModMgr_Runnable_sys/18(EcoModForClimaActv_EcoModForClimaActv_write) -> EcoModForClimaActv_EcoModForClimaActv/1
  ClimaModMgr_Runnable_sys/19(EcuKeepActvFromClimaCtrl_EcuKeepActvFromClimaCtrl_write) -> EcuKeepActvFromClimaCtrl_EcuKeepActvFromClimaCtrl/1
  ClimaModMgr_Runnable_sys/20(EngStrtRemActv_EngStrtRemActv_write) -> EngStrtRemActv_EngStrtRemActv/1
  ClimaModMgr_Runnable_sys/21(FltModForHmiFailr_FltModForHmiFailr_write) -> FltModForHmiFailr_FltModForHmiFailr/1
  ClimaModMgr_Runnable_sys/22(HeatrDiByCarMod_HeatrDiByCarMod_write) -> HeatrDiByCarMod_HeatrDiByCarMod/1
  ClimaModMgr_Runnable_sys/23(HvacActrStReq_HvacActrStReq_write) -> HvacActrStReq_HvacActrStReq/1
  ClimaModMgr_Runnable_sys/24(ManModDurgFanOffActv_ManModDurgFanOffActv_write) -> ManModDurgFanOffActv_ManModDurgFanOffActv/1
  ClimaModMgr_Runnable_sys/25(PrkgClimaActv_PrkgClimaActv_write) -> PrkgClimaActv_PrkgClimaActv/1
  ClimaModMgr_Runnable_sys/26(PrkgClimaDiByCarMod_PrkgClimaDiByCarMod_write) -> PrkgClimaDiByCarMod_PrkgClimaDiByCarMod/1
  ClimaModMgr_Runnable_sys/27(VentnForWinOpenGlbActv_VentnForWinOpenGlbActv_write) -> VentnForWinOpenGlbActv_VentnForWinOpenGlbActv/1
  ClimaModMgr_Runnable_sys/28(EcoClimasts_Ngcc_EcoClimasts_write) -> EcoClimasts_Ngcc_EcoClimasts/1
  ClimaModMgr_Runnable_sys/29(MemSaveFlgForEcoClima_MemSaveFlgForEcoClima_write) -> MemSaveFlgForEcoClima_MemSaveFlgForEcoClima/1
  ClimaModMgr_Runnable_sys/30(MemWrForEcoClima_MemWrForEcoClima_write) -> MemWrForEcoClima_MemWrForEcoClima/1
  ClimaModMgr_Runnable/1 -> ClimaModMgr_Runnable_sys/Trigger

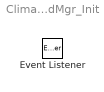
== system ClimaModMgr/ClimaModMgr_Init ==
  Event Listener : EventListener  EventName=reset

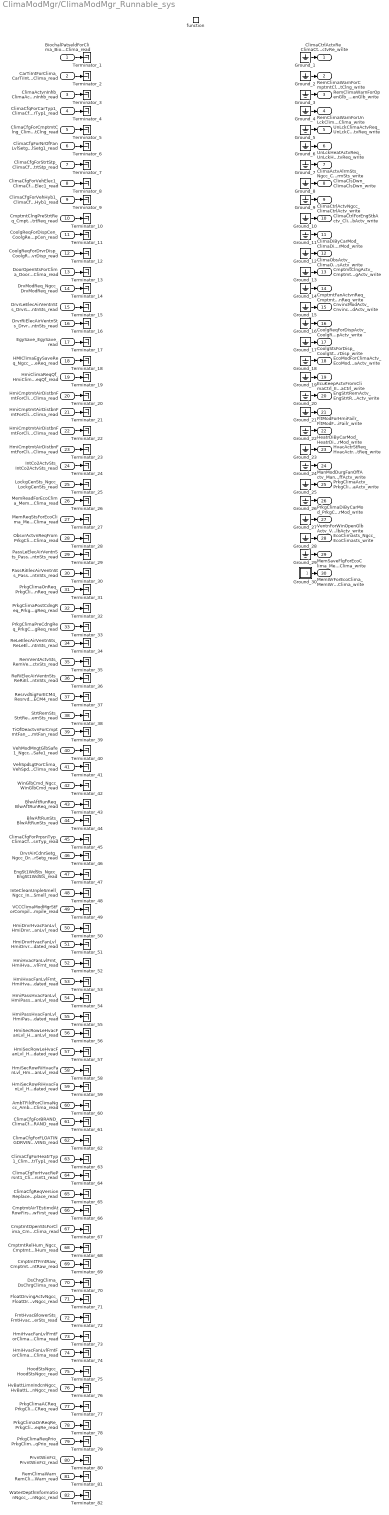
== system ClimaModMgr/ClimaModMgr_Runnable_sys ==
  BiochalPatseldForClima_BiochalPatseldForClima_read : Inport
  CarTiIntForClima_CarTiIntForClima_read : Inport  Port=2
  ClimaActvnInhb_ClimaActvnInhb_read : Inport  Port=3
  ClimaCfgForCarTyp1_ClimaCfgForCarTyp1_read : Inport  Port=4
  ClimaCfgForCmptmtClng_ClimaCfgForCmptmtClng_read : Inport  Port=5
  ClimaCfgForNrOfFanLvlSetg1_ClimaCfgForNrOfFanLvlSetg1_read : Inport  Port=6
  ClimaCfgForStrtStp_ClimaCfgForStrtStp_read : Inport  Port=7
  ClimaCfgForVehElec1_ClimaCfgForVehElec1_read : Inport  Port=8
  ClimaCfgForVehHyb1_ClimaCfgForVehHyb1_read : Inport  Port=9
  CmptmtClngPreStrtReq_CmptmtClngPreStrtReq_read : Inport  Port=10
  CoolgReqForDispCen_CoolgReqForDispCen_read : Inport  Port=11
  CoolgReqForDrvrDisp_CoolgReqForDrvrDisp_read : Inport  Port=12
  DoorOpenStsForClima_DoorOpenStsForClima_read : Inport  Port=13
  DrvModReq_Ngcc_DrvModReq_read : Inport  Port=14
  DrvrLeElecAirVentnSts_DrvrLeElecAirVentnSts_read : Inport  Port=15
  DrvrRiElecAirVentnSts_DrvrRiElecAirVentnSts_read : Inport  Port=16
  EgySave_EgySave_read : Inport  Port=17
  HMIClimaEgySaveReq_Ngcc_HMIClimaEgySaveReq_read : Inport  Port=18
  HmiClimaReqQf_HmiClimaReqQf_read : Inport  Port=19
  HmiCmptmtAirDistbnFrntForClima_HmiCmptmtAirDistbnFrntForClima_read : Inport  Port=20
  HmiCmptmtAirDistbnFrntForClima_HmiCmptmtAirDistbnFrntRiForClima_read : Inport  Port=21
  HmiCmptmtAirDistbnFrntForClima_HmiCmptmtAirDistbnReForClima_read : Inport  Port=22
  HmiCmptmtAirDistbnFrntForClima_HmiCmptmtAirDistbnReRiForClima_read : Inport  Port=23
  IntCo2ActvSts_IntCo2ActvSts_read : Inport  Port=24
  LockgCenSts_Ngcc_LockgCenSts_read : Inport  Port=25
  MemReadForEcoClima_MemReadForEcoClima_read : Inport  Port=26
  MemReqStsForEcoClima_MemReqStsForEcoClima_read : Inport  Port=27
  ObsvrActvnReqFromPrkgClima_ObsvrActvnReqFromPrkgClima_read : Inport  Port=28
  PassLeElecAirVentnSts_PassLeElecAirVentnSts_read : Inport  Port=29
  PassRiElecAirVentnSts_PassRiElecAirVentnSts_read : Inport  Port=30
  PrkgClimaOnReq_PrkgClimaOnReq_read : Inport  Port=31
  PrkgClimaPostCdngReq_PrkgClimaPostCdngReq_read : Inport  Port=32
  PrkgClimaPreCdngReq_PrkgClimaPreCdngReq_read : Inport  Port=33
  ReLeElecAirVentnSts_ReLeElecAirVentnSts_read : Inport  Port=34
  RemVentActvSts_RemVentActvSts_read : Inport  Port=35
  ReRiElecAirVentnSts_ReRiElecAirVentnSts_read : Inport  Port=36
  ResrvdSigForECM4_ResrvdSigForECM4_read : Inport  Port=37
  StrtRemSts_StrtRemSts1WdStrtRemSts_read : Inport  Port=38
  TiOfDeactvnForCmptmtFan_TiOfDeactvnForCmptmtFan_read : Inport  Port=39
  VehModMngtGlbSafe1_Ngcc_VehModMngtGlbSafe1_read : Inport  Port=40
  VehSpdLgtForClima_VehSpdLgtForClima_read : Inport  Port=41
  WinGlbCmd_Ngcc_WinGlbCmd_read : Inport  Port=42
  BlwAftRunReq_BlwAftRunReq_read : Inport  Port=43
  BlwAftRunSts_BlwAftRunSts_read : Inport  Port=44
  ClimaCfgForPrpsnTyp_ClimaCfgForPrpsnTyp_read : Inport  Port=45
  DrvrAirCdnrSetg_Ngcc_DrvrAirCdnrSetg_read : Inport  Port=46
  EngSt1WdSts_Ngcc_EngSt1WdSts_read : Inport  Port=47
  InteCleanUnpleSmell_Ngcc_InteCleanUnpleSmell_read : Inport  Port=48
  VCCClimaModMgrStForCompile_VCCClimaModMgrStForCompile_read : Inport  Port=49
  HmiDrvrHvacFanLvl_HmiDrvrHvacFanLvl_read : Inport  Port=50
  HmiDrvrHvacFanLvl_HmiDrvrHvacFanLvl_IsUpdated_read : Inport  Port=51
  HmiHvacFanLvlFrnt_HmiHvacFanLvlFrnt_read : Inport  Port=52
  HmiHvacFanLvlFrnt_HmiHvacFanLvlFrnt_IsUpdated_read : Inport  Port=53
  HmiPassHvacFanLvl_HmiPassHvacFanLvl_read : Inport  Port=54
  HmiPassHvacFanLvl_HmiPassHvacFanLvl_IsUpdated_read : Inport  Port=55
  HmiSecRowLeHvacFanLvl_HmiSecRowLeHvacFanLvl_read : Inport  Port=56
  HmiSecRowLeHvacFanLvl_HmiSecRowLeHvacFanLvl_IsUpdated_read : Inport  Port=57
  HmiSecRowRiHvacFanLvl_HmiSecRowRiHvacFanLvl_read : Inport  Port=58
  HmiSecRowRiHvacFanLvl_HmiSecRowRiHvacFanLvl_IsUpdated_read : Inport  Port=59
  AmbTFildForClimaNgcc_AmbTFildForClima_read : Inport  Port=60
  ClimaCfgForBRAND_ClimaCfgForBRAND_read : Inport  Port=61
  ClimaCfgForFLOATINGDRVING_ClimaCfgForFLOATINGDRVING_read : Inport  Port=62
  ClimaCfgForHeatrTyp1_ClimaCfgForHeatrTyp1_read : Inport  Port=63
  ClimaCfgForHvacRePrsnt1_ClimaCfgForHvacRePrsnt1_read : Inport  Port=64
  ClimaCfgReqVersionReplace_ClimaCfgReqVersionReplace_read : Inport  Port=65
  CmptmtAirTEstimdAtRowFirst_CmptmtAirTEstimdAtRowFirst_read : Inport  Port=66
  CmptmtOpenStsForClima_CmptmtOpenStsForClima_read : Inport  Port=67
  CmptmtRelHum_Ngcc_CmptmtRelHum_read : Inport  Port=68
  CmptmtTFrntRaw_CmptmtTFrntRaw_read : Inport  Port=69
  DsChrgClima_DsChrgClima_read : Inport  Port=70
  FloatDrvingActvNgcc_FloatDrvingActvNgcc_read : Inport  Port=71
  FrntHvacBlowerSts_FrntHvacBlowerSts_read : Inport  Port=72
  HmiHvacFanLvlFrntForClima_HmiHvacFanLvlFrntForClima_read : Inport  Port=73
  HmiHvacFanLvlFrntForClima_HmiHvacFanLvlFrntRiForClima_read : Inport  Port=74
  HoodStsNgcc_HoodStsNgcc_read : Inport  Port=75
  HvBattLimnIndcnNgcc_HvBattLimnIndcnNgcc_read : Inport  Port=76
  PrkgClimaACReq_PrkgClimaACReq_read : Inport  Port=77
  PrkgClimaOnReqRe_PrkgClimaOnReqRe_read : Inport  Port=78
  PrkgClimaReqPrio_PrkgClimaReqPrio_read : Inport  Port=79
  PrvntWinFrz_PrvntWinFrz_read : Inport  Port=80
  RemClimaWarn_RemClimaWarn_read : Inport  Port=81
  WaterDepthInformationNgcc_WaterDepthInformationNgcc_read : Inport  Port=82
  function : TriggerPort  TriggerType=function-call  VariantControl=(inherit)  SampleTimeType=periodic  SampleTime=-1
  Ground_{1..29} (29 alike) : Ground
  Ground_30 : Subsystem
  Terminator_{1..82} (82 alike) : Terminator
  ClimaCtrlActvRe_ClimaCtrlActvRe_write : Outport
      settings: EnsureOutportIsVirtual=on
  RemClimaWarnForCmptmtClng_RemClimaWarnForCmptmtClng_write : Outport  Port=2
      settings: EnsureOutportIsVirtual=on
  RemClimaWarnForOpenGlb_RemClimaWarnForOpenGlb_write : Outport  Port=3
      settings: EnsureOutportIsVirtual=on
  RemClimaWarnForUnLckClima_RemClimaWarnForUnLckClima_write : Outport  Port=4
      settings: EnsureOutportIsVirtual=on
  UnLckClimaActvReq_UnLckClimaActvReq_write : Outport  Port=5
      settings: EnsureOutportIsVirtual=on
  UnLckHeatActvReq_UnLckHeatActvReq_write : Outport  Port=6
      settings: EnsureOutportIsVirtual=on
  ClimaActvAlrmSts_Ngcc_ClimaActvAlrmSts_write : Outport  Port=7
      settings: EnsureOutportIsVirtual=on
  ClimaClsDwn_ClimaClsDwn_write : Outport  Port=8
      settings: EnsureOutportIsVirtual=on
  ClimaCtrlActvNgcc_ClimaCtrlActv_write : Outport  Port=9
      settings: EnsureOutportIsVirtual=on
  ClimaCtrlForEngStbActv_ClimaCtrlForEngStbActv_write : Outport  Port=10
      settings: EnsureOutportIsVirtual=on
  ClimaDiByCarMod_ClimaDiByCarMod_write : Outport  Port=11
      settings: EnsureOutportIsVirtual=on
  ClimaObsActv_ClimaObsActv_write : Outport  Port=12
      settings: EnsureOutportIsVirtual=on
  CmptmtClngActv_CmptmtClngActv_write : Outport  Port=13
      settings: EnsureOutportIsVirtual=on
  CmptmtFanActvnReq_CmptmtFanActvnReq_write : Outport  Port=14
      settings: EnsureOutportIsVirtual=on
  CnvincModActv_CnvincModActv_write : Outport  Port=15
      settings: EnsureOutportIsVirtual=on
  CoolgReqForDispActv_CoolgReqForDispActv_write : Outport  Port=16
      settings: EnsureOutportIsVirtual=on
  CoolgStsForDisp_CoolgStsForDisp_write : Outport  Port=17
      settings: EnsureOutportIsVirtual=on
  EcoModForClimaActv_EcoModForClimaActv_write : Outport  Port=18
      settings: EnsureOutportIsVirtual=on
  EcuKeepActvFromClimaCtrl_EcuKeepActvFromClimaCtrl_write : Outport  Port=19
      settings: EnsureOutportIsVirtual=on
  EngStrtRemActv_EngStrtRemActv_write : Outport  Port=20
      settings: EnsureOutportIsVirtual=on
  FltModForHmiFailr_FltModForHmiFailr_write : Outport  Port=21
      settings: EnsureOutportIsVirtual=on
  HeatrDiByCarMod_HeatrDiByCarMod_write : Outport  Port=22
      settings: EnsureOutportIsVirtual=on
  HvacActrStReq_HvacActrStReq_write : Outport  Port=23
      settings: EnsureOutportIsVirtual=on
  ManModDurgFanOffActv_ManModDurgFanOffActv_write : Outport  Port=24
      settings: EnsureOutportIsVirtual=on
  PrkgClimaActv_PrkgClimaActv_write : Outport  Port=25
      settings: EnsureOutportIsVirtual=on
  PrkgClimaDiByCarMod_PrkgClimaDiByCarMod_write : Outport  Port=26
      settings: EnsureOutportIsVirtual=on
  VentnForWinOpenGlbActv_VentnForWinOpenGlbActv_write : Outport  Port=27
      settings: EnsureOutportIsVirtual=on
  EcoClimasts_Ngcc_EcoClimasts_write : Outport  Port=28
      settings: EnsureOutportIsVirtual=on
  MemSaveFlgForEcoClima_MemSaveFlgForEcoClima_write : Outport  Port=29
      settings: EnsureOutportIsVirtual=on
  MemWrForEcoClima_MemWrForEcoClima_write : Outport  Port=30
      settings: EnsureOutportIsVirtual=on
  wires:
  BiochalPatseldForClima_BiochalPatseldForClima_read/1 -> Terminator_1/1
  CarTiIntForClima_CarTiIntForClima_read/1 -> Terminator_2/1
  ClimaActvnInhb_ClimaActvnInhb_read/1 -> Terminator_3/1
  ClimaCfgForCarTyp1_ClimaCfgForCarTyp1_read/1 -> Terminator_4/1
  ClimaCfgForCmptmtClng_ClimaCfgForCmptmtClng_read/1 -> Terminator_5/1
  ClimaCfgForNrOfFanLvlSetg1_ClimaCfgForNrOfFanLvlSetg1_read/1 -> Terminator_6/1
  ClimaCfgForStrtStp_ClimaCfgForStrtStp_read/1 -> Terminator_7/1
  ClimaCfgForVehElec1_ClimaCfgForVehElec1_read/1 -> Terminator_8/1
  ClimaCfgForVehHyb1_ClimaCfgForVehHyb1_read/1 -> Terminator_9/1
  CmptmtClngPreStrtReq_CmptmtClngPreStrtReq_read/1 -> Terminator_10/1
  CoolgReqForDispCen_CoolgReqForDispCen_read/1 -> Terminator_11/1
  CoolgReqForDrvrDisp_CoolgReqForDrvrDisp_read/1 -> Terminator_12/1
  DoorOpenStsForClima_DoorOpenStsForClima_read/1 -> Terminator_13/1
  DrvModReq_Ngcc_DrvModReq_read/1 -> Terminator_14/1
  DrvrLeElecAirVentnSts_DrvrLeElecAirVentnSts_read/1 -> Terminator_15/1
  DrvrRiElecAirVentnSts_DrvrRiElecAirVentnSts_read/1 -> Terminator_16/1
  EgySave_EgySave_read/1 -> Terminator_17/1
  HMIClimaEgySaveReq_Ngcc_HMIClimaEgySaveReq_read/1 -> Terminator_18/1
  HmiClimaReqQf_HmiClimaReqQf_read/1 -> Terminator_19/1
  HmiCmptmtAirDistbnFrntForClima_HmiCmptmtAirDistbnFrntForClima_read/1 -> Terminator_20/1
  HmiCmptmtAirDistbnFrntForClima_HmiCmptmtAirDistbnFrntRiForClima_read/1 -> Terminator_21/1
  HmiCmptmtAirDistbnFrntForClima_HmiCmptmtAirDistbnReForClima_read/1 -> Terminator_22/1
  HmiCmptmtAirDistbnFrntForClima_HmiCmptmtAirDistbnReRiForClima_read/1 -> Terminator_23/1
  IntCo2ActvSts_IntCo2ActvSts_read/1 -> Terminator_24/1
  LockgCenSts_Ngcc_LockgCenSts_read/1 -> Terminator_25/1
  MemReadForEcoClima_MemReadForEcoClima_read/1 -> Terminator_26/1
  MemReqStsForEcoClima_MemReqStsForEcoClima_read/1 -> Terminator_27/1
  ObsvrActvnReqFromPrkgClima_ObsvrActvnReqFromPrkgClima_read/1 -> Terminator_28/1
  PassLeElecAirVentnSts_PassLeElecAirVentnSts_read/1 -> Terminator_29/1
  PassRiElecAirVentnSts_PassRiElecAirVentnSts_read/1 -> Terminator_30/1
  PrkgClimaOnReq_PrkgClimaOnReq_read/1 -> Terminator_31/1
  PrkgClimaPostCdngReq_PrkgClimaPostCdngReq_read/1 -> Terminator_32/1
  PrkgClimaPreCdngReq_PrkgClimaPreCdngReq_read/1 -> Terminator_33/1
  ReLeElecAirVentnSts_ReLeElecAirVentnSts_read/1 -> Terminator_34/1
  RemVentActvSts_RemVentActvSts_read/1 -> Terminator_35/1
  ReRiElecAirVentnSts_ReRiElecAirVentnSts_read/1 -> Terminator_36/1
  ResrvdSigForECM4_ResrvdSigForECM4_read/1 -> Terminator_37/1
  StrtRemSts_StrtRemSts1WdStrtRemSts_read/1 -> Terminator_38/1
  TiOfDeactvnForCmptmtFan_TiOfDeactvnForCmptmtFan_read/1 -> Terminator_39/1
  VehModMngtGlbSafe1_Ngcc_VehModMngtGlbSafe1_read/1 -> Terminator_40/1
  VehSpdLgtForClima_VehSpdLgtForClima_read/1 -> Terminator_41/1
  WinGlbCmd_Ngcc_WinGlbCmd_read/1 -> Terminator_42/1
  BlwAftRunReq_BlwAftRunReq_read/1 -> Terminator_43/1
  BlwAftRunSts_BlwAftRunSts_read/1 -> Terminator_44/1
  ClimaCfgForPrpsnTyp_ClimaCfgForPrpsnTyp_read/1 -> Terminator_45/1
  DrvrAirCdnrSetg_Ngcc_DrvrAirCdnrSetg_read/1 -> Terminator_46/1
  EngSt1WdSts_Ngcc_EngSt1WdSts_read/1 -> Terminator_47/1
  InteCleanUnpleSmell_Ngcc_InteCleanUnpleSmell_read/1 -> Terminator_48/1
  VCCClimaModMgrStForCompile_VCCClimaModMgrStForCompile_read/1 -> Terminator_49/1
  HmiDrvrHvacFanLvl_HmiDrvrHvacFanLvl_read/1 -> Terminator_50/1
  HmiDrvrHvacFanLvl_HmiDrvrHvacFanLvl_IsUpdated_read/1 -> Terminator_51/1
  HmiHvacFanLvlFrnt_HmiHvacFanLvlFrnt_read/1 -> Terminator_52/1
  HmiHvacFanLvlFrnt_HmiHvacFanLvlFrnt_IsUpdated_read/1 -> Terminator_53/1
  HmiPassHvacFanLvl_HmiPassHvacFanLvl_read/1 -> Terminator_54/1
  HmiPassHvacFanLvl_HmiPassHvacFanLvl_IsUpdated_read/1 -> Terminator_55/1
  HmiSecRowLeHvacFanLvl_HmiSecRowLeHvacFanLvl_read/1 -> Terminator_56/1
  HmiSecRowLeHvacFanLvl_HmiSecRowLeHvacFanLvl_IsUpdated_read/1 -> Terminator_57/1
  HmiSecRowRiHvacFanLvl_HmiSecRowRiHvacFanLvl_read/1 -> Terminator_58/1
  HmiSecRowRiHvacFanLvl_HmiSecRowRiHvacFanLvl_IsUpdated_read/1 -> Terminator_59/1
  AmbTFildForClimaNgcc_AmbTFildForClima_read/1 -> Terminator_60/1
  ClimaCfgForBRAND_ClimaCfgForBRAND_read/1 -> Terminator_61/1
  ClimaCfgForFLOATINGDRVING_ClimaCfgForFLOATINGDRVING_read/1 -> Terminator_62/1
  ClimaCfgForHeatrTyp1_ClimaCfgForHeatrTyp1_read/1 -> Terminator_63/1
  ClimaCfgForHvacRePrsnt1_ClimaCfgForHvacRePrsnt1_read/1 -> Terminator_64/1
  ClimaCfgReqVersionReplace_ClimaCfgReqVersionReplace_read/1 -> Terminator_65/1
  CmptmtAirTEstimdAtRowFirst_CmptmtAirTEstimdAtRowFirst_read/1 -> Terminator_66/1
  CmptmtOpenStsForClima_CmptmtOpenStsForClima_read/1 -> Terminator_67/1
  CmptmtRelHum_Ngcc_CmptmtRelHum_read/1 -> Terminator_68/1
  CmptmtTFrntRaw_CmptmtTFrntRaw_read/1 -> Terminator_69/1
  DsChrgClima_DsChrgClima_read/1 -> Terminator_70/1
  FloatDrvingActvNgcc_FloatDrvingActvNgcc_read/1 -> Terminator_71/1
  FrntHvacBlowerSts_FrntHvacBlowerSts_read/1 -> Terminator_72/1
  HmiHvacFanLvlFrntForClima_HmiHvacFanLvlFrntForClima_read/1 -> Terminator_73/1
  HmiHvacFanLvlFrntForClima_HmiHvacFanLvlFrntRiForClima_read/1 -> Terminator_74/1
  HoodStsNgcc_HoodStsNgcc_read/1 -> Terminator_75/1
  HvBattLimnIndcnNgcc_HvBattLimnIndcnNgcc_read/1 -> Terminator_76/1
  PrkgClimaACReq_PrkgClimaACReq_read/1 -> Terminator_77/1
  PrkgClimaOnReqRe_PrkgClimaOnReqRe_read/1 -> Terminator_78/1
  PrkgClimaReqPrio_PrkgClimaReqPrio_read/1 -> Terminator_79/1
  PrvntWinFrz_PrvntWinFrz_read/1 -> Terminator_80/1
  RemClimaWarn_RemClimaWarn_read/1 -> Terminator_81/1
  WaterDepthInformationNgcc_WaterDepthInformationNgcc_read/1 -> Terminator_82/1
  Ground_1/1 -> ClimaCtrlActvRe_ClimaCtrlActvRe_write/1
  Ground_2/1 -> RemClimaWarnForCmptmtClng_RemClimaWarnForCmptmtClng_write/1
  Ground_3/1 -> RemClimaWarnForOpenGlb_RemClimaWarnForOpenGlb_write/1
  Ground_4/1 -> RemClimaWarnForUnLckClima_RemClimaWarnForUnLckClima_write/1
  Ground_5/1 -> UnLckClimaActvReq_UnLckClimaActvReq_write/1
  Ground_6/1 -> UnLckHeatActvReq_UnLckHeatActvReq_write/1
  Ground_7/1 -> ClimaActvAlrmSts_Ngcc_ClimaActvAlrmSts_write/1
  Ground_8/1 -> ClimaClsDwn_ClimaClsDwn_write/1
  Ground_9/1 -> ClimaCtrlActvNgcc_ClimaCtrlActv_write/1
  Ground_10/1 -> ClimaCtrlForEngStbActv_ClimaCtrlForEngStbActv_write/1
  Ground_11/1 -> ClimaDiByCarMod_ClimaDiByCarMod_write/1
  Ground_12/1 -> ClimaObsActv_ClimaObsActv_write/1
  Ground_13/1 -> CmptmtClngActv_CmptmtClngActv_write/1
  Ground_14/1 -> CmptmtFanActvnReq_CmptmtFanActvnReq_write/1
  Ground_15/1 -> CnvincModActv_CnvincModActv_write/1
  Ground_16/1 -> CoolgReqForDispActv_CoolgReqForDispActv_write/1
  Ground_17/1 -> CoolgStsForDisp_CoolgStsForDisp_write/1
  Ground_18/1 -> EcoModForClimaActv_EcoModForClimaActv_write/1
  Ground_19/1 -> EcuKeepActvFromClimaCtrl_EcuKeepActvFromClimaCtrl_write/1
  Ground_20/1 -> EngStrtRemActv_EngStrtRemActv_write/1
  Ground_21/1 -> FltModForHmiFailr_FltModForHmiFailr_write/1
  Ground_22/1 -> HeatrDiByCarMod_HeatrDiByCarMod_write/1
  Ground_23/1 -> HvacActrStReq_HvacActrStReq_write/1
  Ground_24/1 -> ManModDurgFanOffActv_ManModDurgFanOffActv_write/1
  Ground_25/1 -> PrkgClimaActv_PrkgClimaActv_write/1
  Ground_26/1 -> PrkgClimaDiByCarMod_PrkgClimaDiByCarMod_write/1
  Ground_27/1 -> VentnForWinOpenGlbActv_VentnForWinOpenGlbActv_write/1
  Ground_28/1 -> EcoClimasts_Ngcc_EcoClimasts_write/1
  Ground_29/1 -> MemSaveFlgForEcoClima_MemSaveFlgForEcoClima_write/1
  Ground_30/1(Out1) -> MemWrForEcoClima_MemWrForEcoClima_write/1

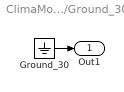
== system ClimaModMgr/ClimaModMgr_Runnable_sys/Ground_30 ==
  Ground_30 : Ground
  Out1 : Outport  OutDataTypeStr=Bus: MemWrForEcoClima  PortDimensions=1
  wires:
  Ground_30/1 -> Out1/1
